simulink model "l64_clean"  (Simulink 7.9)
settings: Solver=ode45  StartTime=0.0  StopTime=1500000  RelTol=1e-3
undefined symbols (not defined in the file): pfb_coeff_gen_calc, sfp
libraries: xbsIndex_r4 x22038, casper_library_misc x643, casper_library_delays x214, xps_library x24, xbsReplacements_r4 x16, casper_library_pfbs x16, casper_library_ffts_twiddle x8, simulink x1
(part 1 of 317)
settings Model: Creator=jkocz  LastModifiedBy=jkocz
settings DebuggingCC: FcnCallInpInsideContextMsg=Use local settings  StrictBusMsg=Warning
settings GRTTargetCC: CombineOutputUpdateFcns=off  GRTInterface=on

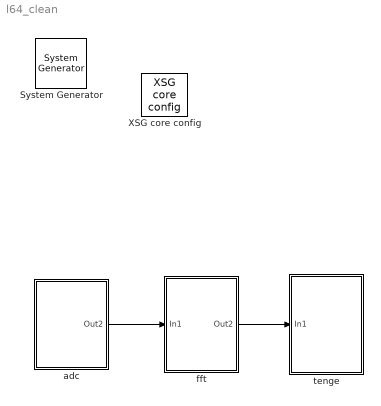
== system l64_clean ==
  " System Generator" : xbsIndex_r4/ System Generator  Tag=genX  xilinxfamily=virtex6  part=xc6vsx475t  speed=-1  package=ff1759  synthesis_tool=XST  clock_wrapper=Clock Enables  directory=./l64_clean/sysgen  proj_type=Project Navigator  Synth_file=XST Defaults  Impl_file=ISE Defaults  testbench=off  simulink_period=1  sysclk_period=5  dcm_input_clock_period=100  incr_netlist=off  trim_vbits=Everywhere in SubSystem  dbl_ovrd=According to Block Masks  core_generation=According to Block Masks  run_coregen=off  deprecated_control=off  eval_field=0  block_type=sysgen
      settings: block_version=11.4
  XSG core config : xps_library/XSG core config  Tag=xps:xsg  hw_sys=ROACH2:sx475t  clk_src=adc0_clk  ROACH_clk_src=adc0_clk  ROACH2_clk_src=adc0_clk  clk_rate=200  sample_period=1  synthesis_tool=XST
  adc : Subsystem
  fft : Subsystem
  tenge : Subsystem
  wires:
  adc/1(Out2) -> fft/1(In1)
  fft/1(Out2) -> tenge/1(In1)

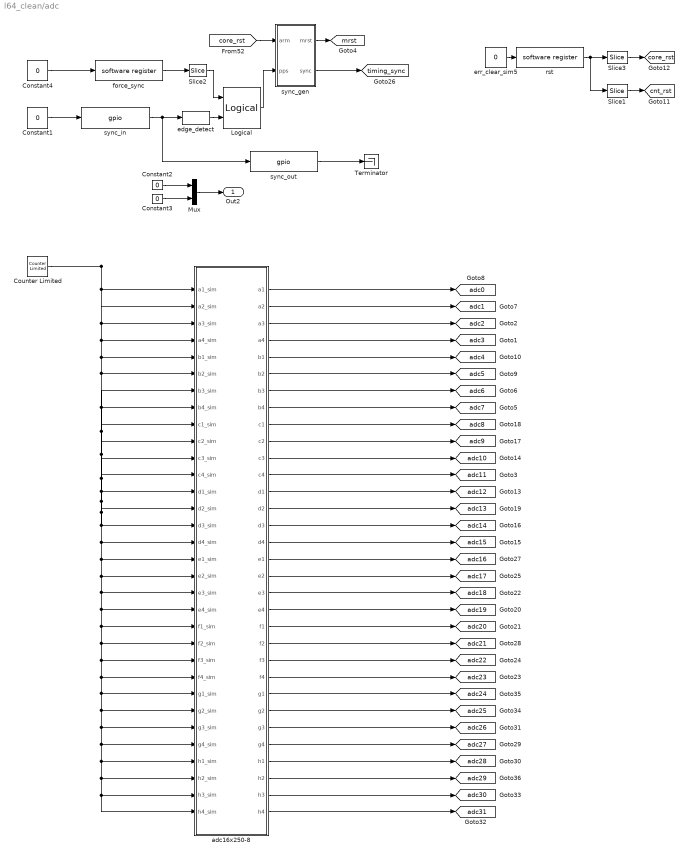
== system l64_clean/adc ==
  Constant1 : Constant  Value=0  SampleTime=1/4
  Constant2 : Constant  Value=0
  Constant3 : Constant  Value=0
  Constant4 : Constant  Value=0  SampleTime=1/4
  Counter Limited : simulink/Sources/Counter Limited  uplimit=4095  tsamp=-1
  Logical : xbsIndex_r4/Logical  logical_function=OR  inputs=2  en=off  latency=0  precision=Full  arith_type=Unsigned  n_bits=16  bin_pt=0  align_bp=on  dbl_ovrd=off  block_type=logical
  Mux : Mux  Inputs=2
  Slice1 : xbsIndex_r4/Slice  nbits=1  boolean_output=on  mode=Lower Bit Location + Width  bit1=0  base1=MSB of Input  bit0=0  base0=LSB of Input  dbl_ovrd=off  block_type=slice
      settings: block_version=10.1.3
  Slice2 : xbsIndex_r4/Slice  nbits=1  boolean_output=on  mode=Lower Bit Location + Width  bit1=0  base1=MSB of Input  bit0=0  base0=LSB of Input  dbl_ovrd=off  block_type=slice
      settings: block_version=10.1.3
  Slice3 : xbsIndex_r4/Slice  nbits=1  boolean_output=on  mode=Lower Bit Location + Width  bit1=0  base1=MSB of Input  bit0=1  base0=LSB of Input  dbl_ovrd=off  block_type=slice
      settings: block_version=10.1.3
  Terminator : Terminator
  adc16x250-8 : Subsystem  Tag=xps:adc16  AncestorBlock=xps_library/ADCs/adc16x250-8  mask(ADC16){board_count=2, roach2_rev=2, zdok_rev=1}
      mask prompts: board_count "Number of ADC16 Boards"; roach2_rev "ROACH2 Revision"; zdok_rev "ZDOK Pinout Revision"
      mask help: 16-input ADC. When using one ADC16 board, it must be in ZDOK0.
      mask init:
      | % Only board_count is needed by init function
      | adc16_init(gcb, ...
      |     'board_count', board_count);
      settings: AttributesFormatString=r2 rev%<roach2_rev>\nzdok rev%<zdok_rev>
      mask Help:
      | This is the CASPER "yellow block" for the CASPER ADC16 board.  Each ADC
      | board has four ADC chips.  Each ADC chip accepts four inputs.  The four
      | 8-bit outputs from each ADC chip are concatenated into a 32-bit signal.
      mask StyleString: popup(1|2),popup(1|2),popup(1|2)
  edge_detect : casper_library_misc/edge_detect  edge=Rising  polarity=Active High  x_in=[0 0.08 0.08 0.16 0.16 0.24 0.24 0.32 0.32 0.4]  y_in="[-1 -1  1  1  1  1  1  1  1  1]"  x_out=[0.6 0.68 0.68 0.76 0.76 0.84 0.84 0.92 0.92 1]  y_out="[-1 -1  1  1 -1 -1 -1 -1 -1 -1]"
  err_clear_sim5 : Constant  Value=0
  force_sync : xps_library/software register  Tag=xps:sw_reg  io_dir=From Processor  arith_type=Unsigned  bitwidth=32  bin_pt=0  sample_period=1  gw_name=l64_clean_adc_force_sync_user_data_out
      link override r_10gbe_demo_gbe_core_rst_user_data_out: arith_type=Unsigned  n_bits=32  bin_pt=0  period=1
      link override r_10gbe_test_cnt_rst_user_data_out: arith_type=Unsigned  n_bits=32  bin_pt=0  period=1
      link override r_10gbe_test_gbe_core_rst_user_data_out: arith_type=Unsigned  n_bits=32  bin_pt=0  period=1
      link override r_xaui_test_cnt_rst_user_data_out: arith_type=Unsigned  n_bits=32  bin_pt=0  period=1
  rst : xps_library/software register  Tag=xps:sw_reg  io_dir=From Processor  arith_type=Unsigned  bitwidth=32  bin_pt=0  sample_period=1  gw_name=l64_clean_adc_rst_user_data_out
      link override r_10gbe_demo_gbe_core_rst_user_data_out: arith_type=Unsigned  n_bits=32  bin_pt=0  period=1
      link override r_10gbe_test_cnt_rst_user_data_out: arith_type=Unsigned  n_bits=32  bin_pt=0  period=1
      link override r_10gbe_test_gbe_core_rst_user_data_out: arith_type=Unsigned  n_bits=32  bin_pt=0  period=1
      link override r_xaui_test_cnt_rst_user_data_out: arith_type=Unsigned  n_bits=32  bin_pt=0  period=1
  sync_gen : Subsystem  mask(sync_gen_hw){sync_period_bits=27}
      mask prompts: sync_period_bits "Size of sync generator counter (bits)"
      mask help:
      | This circuit implements a scheme for synchronizing a design to within an FPGA clock of an external 1 pulse-per-second signal.
      |
      | * An arm pulse will cause the sync_gen to stop issuing sync pulses.
      | * It will then ignore the next input trigger (1PPS), while sync is flushed through the DSP pipeline.
      | * It will then lock onto the next trigger (1PPS) and issue syncs thereafter every sync_period clocks.
      | * You will need a multiple of (pfb_fir taps*LCM(FFT reorder orders) *fft bins/parallel inputs). See the sync memo for further details.
  sync_in : xps_library/gpio  Tag=xps:gpio  io_group=ROACH2:sync_in  io_dir=in  arith_type=Boolean  bitwidth=1  bin_pt=0  bit_index=0  sample_period=1  use_single_ended=off  use_ddr=off  reg_iob=on  reg_clk_phase=0  termination=None
  sync_out : xps_library/gpio  Tag=xps:gpio  io_group=ROACH2:sync_out  io_dir=out  arith_type=Boolean  bitwidth=1  bin_pt=0  bit_index=0  sample_period=1  use_single_ended=off  use_ddr=off  reg_iob=on  reg_clk_phase=0  termination=None
  Out2 : Outport
  tag scope: adc0=global, adc10=global, adc11=global, adc12=global, adc13=global, adc14=global, adc15=global, adc16=global, adc17=global, adc18=global, adc19=global, adc1=global, adc20=global, adc21=global, adc22=global, adc23=global, adc24=global, adc25=global, adc26=global, adc27=global, adc28=global, adc29=global, adc2=global, adc30=global, adc31=global, adc3=global, adc4=global, adc5=global, adc6=global, adc7=global, adc8=global, adc9=global, cnt_rst=global, core_rst=global, mrst=global, timing_sync=global
  wires:
  sync_gen/1(mrst) -> [mrst]
  sync_gen/2(sync) -> [timing_sync]
  [core_rst] -> sync_gen/1(arm)
  Mux/1 -> Out2/1
  Constant2/1 -> Mux/1
  Constant3/1 -> Mux/2
  err_clear_sim5/1 -> rst/1
  rst/1 -> Slice3/1, Slice1/1
  Slice1/1 -> [cnt_rst]
  Slice3/1 -> [core_rst]
  force_sync/1 -> Slice2/1
  Constant4/1 -> force_sync/1
  adc16x250-8/1(a1) -> [adc0]
  Counter Limited/1 -> adc16x250-8/31(h3_sim), adc16x250-8/32(h4_sim), adc16x250-8/30(h2_sim), adc16x250-8/29(h1_sim), adc16x250-8/28(g4_sim), adc16x250-8/27(g3_sim), adc16x250-8/26(g2_sim), adc16x250-8/25(g1_sim), adc16x250-8/24(f4_sim), adc16x250-8/23(f3_sim), adc16x250-8/22(f2_sim), adc16x250-8/21(f1_sim), adc16x250-8/20(e4_sim), adc16x250-8/19(e3_sim), adc16x250-8/18(e2_sim), adc16x250-8/17(e1_sim), adc16x250-8/16(d4_sim), adc16x250-8/15(d3_sim), adc16x250-8/14(d2_sim), adc16x250-8/13(d1_sim), adc16x250-8/12(c4_sim), adc16x250-8/11(c3_sim), adc16x250-8/10(c2_sim), adc16x250-8/9(c1_sim), adc16x250-8/8(b4_sim), adc16x250-8/7(b3_sim), adc16x250-8/6(b2_sim), adc16x250-8/5(b1_sim), adc16x250-8/4(a4_sim), adc16x250-8/3(a3_sim), adc16x250-8/2(a2_sim), adc16x250-8/1(a1_sim)
  adc16x250-8/2(a2) -> [adc1]
  adc16x250-8/3(a3) -> [adc2]
  adc16x250-8/4(a4) -> [adc3]
  adc16x250-8/5(b1) -> [adc4]
  adc16x250-8/6(b2) -> [adc5]
  adc16x250-8/7(b3) -> [adc6]
  adc16x250-8/8(b4) -> [adc7]
  adc16x250-8/9(c1) -> [adc8]
  adc16x250-8/10(c2) -> [adc9]
  adc16x250-8/11(c3) -> [adc10]
  adc16x250-8/12(c4) -> [adc11]
  adc16x250-8/13(d1) -> [adc12]
  adc16x250-8/14(d2) -> [adc13]
  adc16x250-8/15(d3) -> [adc14]
  adc16x250-8/16(d4) -> [adc15]
  adc16x250-8/17(e1) -> [adc16]
  adc16x250-8/18(e2) -> [adc17]
  adc16x250-8/19(e3) -> [adc18]
  adc16x250-8/20(e4) -> [adc19]
  adc16x250-8/21(f1) -> [adc20]
  adc16x250-8/22(f2) -> [adc21]
  adc16x250-8/23(f3) -> [adc22]
  adc16x250-8/24(f4) -> [adc23]
  adc16x250-8/25(g1) -> [adc24]
  adc16x250-8/26(g2) -> [adc25]
  adc16x250-8/27(g3) -> [adc26]
  adc16x250-8/28(g4) -> [adc27]
  adc16x250-8/29(h1) -> [adc28]
  adc16x250-8/30(h2) -> [adc29]
  adc16x250-8/31(h3) -> [adc30]
  adc16x250-8/32(h4) -> [adc31]
  Constant1/1 -> sync_in/1
  Logical/1 -> sync_gen/2(pps)
  sync_out/1 -> Terminator/1
  edge_detect/1 -> Logical/2
  Slice2/1 -> Logical/1
  sync_in/1 -> edge_detect/1, sync_out/1

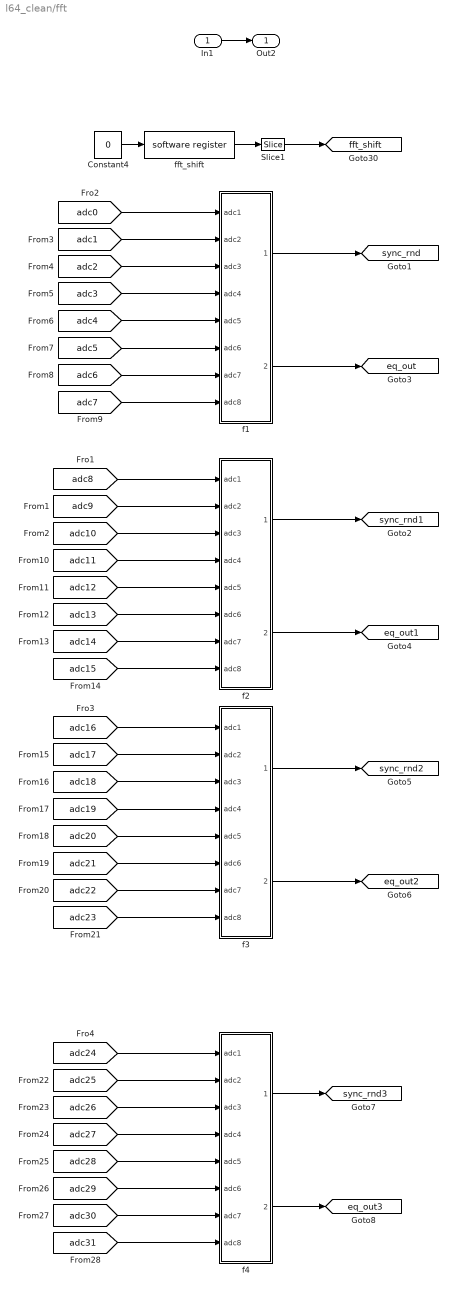
== system l64_clean/fft ==
  In1 : Inport
  Constant4 : Constant  Value=0  SampleTime=1/4
  Slice1 : xbsIndex_r4/Slice  nbits=16  boolean_output=off  mode=Lower Bit Location + Width  bit1=0  base1=MSB of Input  bit0=0  base0=LSB of Input  dbl_ovrd=off  block_type=slice
      settings: block_version=10.1.3
  f1 : Subsystem
  f2 : Subsystem
  f3 : Subsystem
  f4 : Subsystem
  fft_shift : xps_library/software register  Tag=xps:sw_reg  io_dir=From Processor  arith_type=Unsigned  bitwidth=32  bin_pt=0  sample_period=1  gw_name=l64_clean_fft_fft_shift_user_data_out
      link override r_10gbe_demo_gbe_core_rst_user_data_out: arith_type=Unsigned  n_bits=32  bin_pt=0  period=1
      link override r_10gbe_test_cnt_rst_user_data_out: arith_type=Unsigned  n_bits=32  bin_pt=0  period=1
      link override r_10gbe_test_gbe_core_rst_user_data_out: arith_type=Unsigned  n_bits=32  bin_pt=0  period=1
      link override r_xaui_test_cnt_rst_user_data_out: arith_type=Unsigned  n_bits=32  bin_pt=0  period=1
  Out2 : Outport
  tag scope: eq_out1=global, eq_out2=global, eq_out3=global, eq_out=global, fft_shift=global, sync_rnd1=global, sync_rnd2=global, sync_rnd3=global, sync_rnd=global
  wires:
  In1/1 -> Out2/1
  fft_shift/1 -> Slice1/1
  Constant4/1 -> fft_shift/1
  Slice1/1 -> [fft_shift]
  [adc0] -> f1/1(adc1)
  [adc1] -> f1/2(adc2)
  f1/1(sync_rnd) -> [sync_rnd]
  f1/2(eq_out) -> [eq_out]
  [adc2] -> f1/3(adc3)
  [adc3] -> f1/4(adc4)
  [adc4] -> f1/5(adc5)
  [adc5] -> f1/6(adc6)
  [adc6] -> f1/7(adc7)
  [adc7] -> f1/8(adc8)
  f2/1(sync_rnd) -> [sync_rnd1]
  f2/2(eq_out) -> [eq_out1]
  [adc8] -> f2/1(adc1)
  [adc9] -> f2/2(adc2)
  [adc10] -> f2/3(adc3)
  [adc11] -> f2/4(adc4)
  [adc12] -> f2/5(adc5)
  [adc13] -> f2/6(adc6)
  [adc14] -> f2/7(adc7)
  [adc15] -> f2/8(adc8)
  [adc16] -> f3/1(adc1)
  [adc17] -> f3/2(adc2)
  [adc18] -> f3/3(adc3)
  [adc19] -> f3/4(adc4)
  [adc20] -> f3/5(adc5)
  [adc21] -> f3/6(adc6)
  [adc22] -> f3/7(adc7)
  [adc23] -> f3/8(adc8)
  f3/1(sync_rnd) -> [sync_rnd2]
  f3/2(eq_out) -> [eq_out2]
  f4/1(sync_rnd) -> [sync_rnd3]
  f4/2(eq_out) -> [eq_out3]
  [adc24] -> f4/1(adc1)
  [adc25] -> f4/2(adc2)
  [adc26] -> f4/3(adc3)
  [adc27] -> f4/4(adc4)
  [adc28] -> f4/5(adc5)
  [adc29] -> f4/6(adc6)
  [adc30] -> f4/7(adc7)
  [adc31] -> f4/8(adc8)

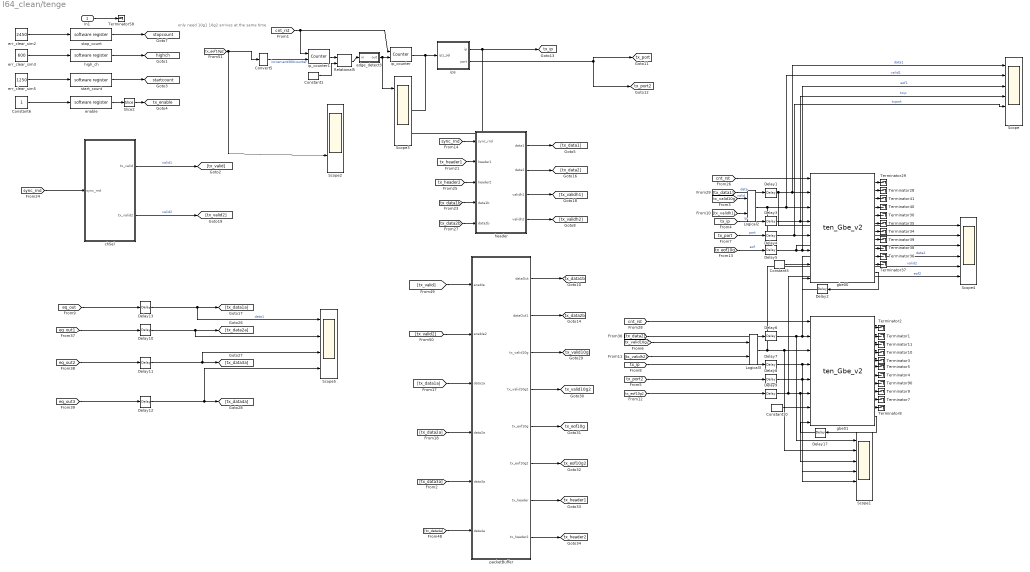
== system l64_clean/tenge ==
  note: only need 10g1
      | 10g2 arrives at the same time
  In1 : Inport
  Constant1 : xbsIndex_r4/Constant  const=1  gui_display_data_type=Fixed-point  arith_type=Unsigned  n_bits=2  bin_pt=0  preci_type=Single  exp_width=8  frac_width=24  explicit_period=on  period=1  equ=P=C  opselect=C  inp2=PCIN>>17  opr=+  inp1=P  carry=CIN  dbl_ovrd=off  block_type=constant
  Constant10 : xbsIndex_r4/Constant  const=1  gui_display_data_type=Fixed-point  arith_type=Boolean  n_bits=1  bin_pt=0  preci_type=Single  exp_width=8  frac_width=24  explicit_period=on  period=1  equ=P=C  opselect=C  inp2=PCIN>>17  opr=+  inp1=P  carry=CIN  dbl_ovrd=off  block_type=constant
  Constant3 : xbsIndex_r4/Constant  const=1  gui_display_data_type=Fixed-point  arith_type=Boolean  n_bits=1  bin_pt=0  preci_type=Single  exp_width=8  frac_width=24  explicit_period=on  period=1  equ=P=C  opselect=C  inp2=PCIN>>17  opr=+  inp1=P  carry=CIN  dbl_ovrd=off  block_type=constant
  Constant6 : Constant
  Convert5 : xbsIndex_r4/Convert  gui_display_data_type=Boolean  arith_type=Boolean  n_bits=64  bin_pt=0  float_type=Single  exp_bits=8  fraction_bits=24  quantization=Truncate  overflow=Wrap  en=off  latency=1  dbl_ovrd=off  pipeline=off  block_type=convert
      settings: block_version=11.4
  Delay{1..4,6..8,17} (8 alike) : xbsIndex_r4/Delay  rst=off  infoeditControl=Selection of Reset will increase slice count due to use of real FFs and instead of SRLs  en=off  latency=1  dbl_ovrd=off  reg_retiming=on  block_type=delay
  Delay{10..13} (4 alike) : xbsIndex_r4/Delay  rst=off  infoeditControl=Selection of Reset will increase slice count due to use of real FFs and instead of SRLs  en=off  latency=5  dbl_ovrd=off  reg_retiming=on  block_type=delay
      settings: xl_area=[0, 0, 0, 0, 0, 0, 0]
  Delay5 : xbsIndex_r4/Delay  rst=off  infoeditControl=Selection of Reset will increase slice count due to use of real FFs and instead of SRLs  en=off  latency=3  dbl_ovrd=off  reg_retiming=on  block_type=delay
  Delay9 : xbsIndex_r4/Delay  rst=off  infoeditControl=Selection of Reset will increase slice count due to use of real FFs and instead of SRLs  en=off  latency=3  dbl_ovrd=off  reg_retiming=on  block_type=delay
  Logical2 : xbsIndex_r4/Logical  logical_function=OR  inputs=2  en=off  latency=3  precision=Full  arith_type=Unsigned  n_bits=16  bin_pt=0  align_bp=on  dbl_ovrd=off  block_type=logical
      settings: block_version=11.4
  Logical3 : xbsIndex_r4/Logical  logical_function=OR  inputs=2  en=off  latency=3  precision=Full  arith_type=Unsigned  n_bits=16  bin_pt=0  align_bp=on  dbl_ovrd=off  block_type=logical
      settings: block_version=11.4
  Relational5 : xbsIndex_r4/Relational  mode=a=b  en=off  latency=1  dbl_ovrd=off  block_type=relational
      settings: xl_area=[0, 0, 0, 0, 0, 0, 0]  block_version=10.1.3
  Scope : Scope  NumInputPorts=6
      settings: TimeRange=125000  YMin=0~0~0~4012~3800.95~-1  YMax=7000~1~1~4022~4201.05~1  SaveName=ScopeData8
  Scope1 : Scope  NumInputPorts=6
      settings: YMin=0~0~0~0~0~-5  YMax=900~1~1~3~30000~5  SaveName=ScopeData9
  Scope2 : Scope  NumInputPorts=2
      settings: YMin=0~0  YMax=7000~1  SaveName=ScopeData7
  Scope3 : Scope  NumInputPorts=3
      settings: YMin=0~0~-5  YMax=7000~1~5  SaveName=ScopeData10
  Scope4 : Scope  NumInputPorts=6
      settings: TimeRange=125000  YMin=0~0~0~4012~3800.95~-1  YMax=7000~1~1~4022~4201.05~1  SaveName=ScopeData11
  Scope5 : Scope  NumInputPorts=4
      settings: YMin=0~0~-5~-5  YMax=7000~1~5~5  SaveName=ScopeData13
  Slice2 : xbsIndex_r4/Slice  nbits=1  boolean_output=off  mode=Lower Bit Location + Width  bit1=1  base1=MSB of Input  bit0=0  base0=LSB of Input  dbl_ovrd=off  block_type=slice
      settings: block_version=10.1.3
  Terminator{1..5,7..11,28..30,34..41,58,90} (23 alike) : Terminator
  chSel : Subsystem
  edge_detect3 : Subsystem  AncestorBlock=casper_library_misc/edge_detect  mask(edge_detect){edge=Rising, polarity=Active High, x_in=[0 0.08 0.08 0.16 0.16 0.24 0.24 0.32 0.32 0.4], y_in=[-1 -1  1  1  1  1  1  1  1  1], x_out=[0.6 0.68 0.68 0.76 0.76 0.84 0.84 0.92 0.92 1], y_out=[-1 -1  1  1 -1 -1 -1 -1 -1 -1]}
      mask prompts: edge "Edge Type"; polarity "Output Polarity"; x_in "Input X Positions"; y_in "Input Y Positions"; x_out "Output X Positions"; y_out "Output Y Positions"
      mask help: Output is active for one-cycle after each edge of the specified type (i.e. rising, falling or both).
      mask init:
      | edge_detect_init(gcb,...
      |     'edge',edge,...
      |     'polarity',polarity,...
      |     'x_in',x_in,...
      |     'x_out',x_out,...
      |     'y_in',y_in,...
      |     'y_out',y_out);
      | 
      | x_in_num=str2num(getfield(getfield(get_param(gcb,'UserData'),'parameters'),'x_in'));
      | x_out_num=str2num(getfield(getfield(get_param(gcb,'UserData'),'parameters'),'x_out'));
      | y_in_num=str2num(getfield(getfield(get_param(gcb,'UserData'),'parameters'),'y_in'));
      | y_out_num=str2num(getfield(getfield(get_param(gcb,'UserData'),'parameters'),'y_out'));
      settings: AttributesFormatString=|
      | rising edges
      | active high
      mask Help: Output is active for one-cycle after each edge of the specified type (i.e. rising, falling, or both).  Latency is zero.
      mask StyleString: popup(Rising|Falling|Both),popup(Active High|Active Low),edit,edit,edit,edit
      mask Display:
      | plot(x_in_num,0.5+y_in_num*0.2);
      | plot(x_out_num,0.5+y_out_num*0.2);
  enable : xps_library/software register  Tag=xps:sw_reg  io_dir=From Processor  arith_type=Unsigned  bitwidth=32  bin_pt=0  sample_period=1  gw_name=l64_clean_tenge_enable_user_data_out
      link override r_10gbe_demo_pkt_sim_run_user_data_out: arith_type=Unsigned  n_bits=32  bin_pt=0  period=1
      link override r_10gbe_test_pkt_sim_run_user_data_out: arith_type=Unsigned  n_bits=32  bin_pt=0  period=1
      link override test_pkt_buffer_10_1_p_mux_up_run_user_data_out: arith_type=Unsigned  n_bits=32  bin_pt=0  period=1
  err_clear_sim2 : Constant  Value=2450
  err_clear_sim3 : Constant  Value=600
  err_clear_sim5 : Constant  Value=1250
  gbe00 : xps_library/ten_Gbe_v2  Tag=xps:tengbe_v2  flavour=sfp+  slot=0  port_r1=0  port_r2_cx4=0  port_r2_sfpp=0  rx_dist_ram=off  large_frames=on  show_param=on  pre_emph_r2=0.74  pre_emph=3  post_emph_r2=0.18  rxeqmix_r2=7  swing_r2=940  swing=800  fab_en=off  fab_mac=hex2dec('123456789100')  fab_ip=192*(2^24) + 168*(2^16) + 5*(2^8) + 14*(2^0)  fab_udp=10002  fab_gate=192*(2^24) + 168*(2^16) + 5*(2^8) + 0  cpu_rx_en=on  cpu_tx_en=on
  gbe01 : xps_library/ten_Gbe_v2  Tag=xps:tengbe_v2  flavour=sfp+  slot=0  port_r1=0  port_r2_cx4=0  port_r2_sfpp=1  rx_dist_ram=off  large_frames=on  show_param=on  pre_emph_r2=0.74  pre_emph=3  post_emph_r2=0.18  rxeqmix_r2=7  swing_r2=940  swing=800  fab_en=off  fab_mac=hex2dec('123456789105')  fab_ip=192*(2^24) + 168*(2^16) + 6*(2^8) + 15*(2^0)  fab_udp=10002  fab_gate=192*(2^24) + 168*(2^16) + 6*(2^8) + 0  cpu_rx_en=on  cpu_tx_en=on
  header : Subsystem
  high_ch : xps_library/software register  Tag=xps:sw_reg  io_dir=From Processor  arith_type=Unsigned  bitwidth=32  bin_pt=0  sample_period=1  gw_name=l64_clean_tenge_high_ch_user_data_out
      link override r_10gbe_demo_dest_ip_user_data_out: arith_type=Unsigned  n_bits=32  bin_pt=0  period=1
      link override r_10gbe_test_dest_ip_user_data_out: arith_type=Unsigned  n_bits=32  bin_pt=0  period=1
      link override r_xaui_test_cnt_rst_user_data_out: arith_type=Unsigned  n_bits=32  bin_pt=0  period=1
  ip_counter : xbsIndex_r4/Counter  cnt_type=Free Running  cnt_to=3  operation=Up  start_count=0  cnt_by_val=1  arith_type=Unsigned  n_bits=2  bin_pt=0  load_pin=off  rst=on  en=on  explicit_period=on  period=1  dbl_ovrd=off  use_behavioral_HDL=off  implementation=Fabric  block_type=counter
      settings: block_version=11.4
  ip_counter1 : xbsIndex_r4/Counter  cnt_type=Free Running  cnt_to=0  operation=Up  start_count=1  cnt_by_val=1  arith_type=Unsigned  n_bits=1  bin_pt=0  load_pin=off  rst=on  en=on  explicit_period=on  period=1  dbl_ovrd=off  use_behavioral_HDL=off  implementation=Fabric  block_type=counter
      settings: block_version=11.4
  ips : Subsystem
  packetBuffer : Subsystem
  start_count : xps_library/software register  Tag=xps:sw_reg  io_dir=From Processor  arith_type=Unsigned  bitwidth=32  bin_pt=0  sample_period=1  gw_name=l64_clean_tenge_start_count_user_data_out
      link override r_10gbe_demo_dest_ip_user_data_out: arith_type=Unsigned  n_bits=32  bin_pt=0  period=1
      link override r_10gbe_test_dest_ip_user_data_out: arith_type=Unsigned  n_bits=32  bin_pt=0  period=1
      link override r_xaui_test_cnt_rst_user_data_out: arith_type=Unsigned  n_bits=32  bin_pt=0  period=1
  stop_count : xps_library/software register  Tag=xps:sw_reg  io_dir=From Processor  arith_type=Unsigned  bitwidth=32  bin_pt=0  sample_period=1  gw_name=l64_clean_tenge_stop_count_user_data_out
      link override leda_64_sim_tenge_stop_count_user_data_out: arith_type=Unsigned  n_bits=32  bin_pt=0  period=1
      link override r_10gbe_demo_dest_ip_user_data_out: arith_type=Unsigned  n_bits=32  bin_pt=0  period=1
      link override r_10gbe_test_dest_ip_user_data_out: arith_type=Unsigned  n_bits=32  bin_pt=0  period=1
      link override r_xaui_test_cnt_rst_user_data_out: arith_type=Unsigned  n_bits=32  bin_pt=0  period=1
  tag scope: highch=global, startcount=global, stopcount=global, tx_data1b=global, tx_data2b=global, tx_enable=global, tx_eof10g2=global, tx_eof10g=global, tx_header1=global, tx_header2=global, tx_ip=global, tx_port2=global, tx_port=global, tx_valid10g2=global, tx_valid10g=global
  wires:
  In1/1 -> Terminator58/1
  err_clear_sim2/1 -> stop_count/1
  stop_count/1 -> [stopcount]
  err_clear_sim3/1 -> high_ch/1
  high_ch/1 -> [highch]
  err_clear_sim5/1 -> start_count/1
  start_count/1 -> [startcount]
  enable/1 -> Slice2/1
  Constant6/1 -> enable/1
  Slice2/1 -> [tx_enable]
  [sync_rnd] -> chSel/1(sync_rnd)
  gbe00/12 -> Delay2/1
  Delay2/1 -> gbe00/8
  gbe00/5 -> Terminator30/1
  gbe00/4 -> Terminator40/1
  gbe00/9 -> Terminator38/1
  gbe00/1 -> Terminator29/1
  gbe00/2 -> Terminator28/1
  gbe00/3 -> Terminator41/1
  gbe00/6 -> Terminator35/1
  gbe00/7 -> Terminator34/1
  gbe00/8 -> Terminator39/1
  gbe00/10 -> Terminator36/1
  gbe00/11 -> Terminator37/1
  [cnt_rst] -> gbe00/1
  Constant10/1 -> gbe01/7
  gbe01/12 -> Delay17/1
  Delay17/1 -> gbe01/8
  gbe01/5 -> Terminator3/1
  gbe01/4 -> Terminator10/1
  gbe01/9 -> Terminator9/1
  gbe01/1 -> Terminator2/1
  gbe01/2 -> Terminator1/1
  gbe01/3 -> Terminator11/1
  gbe01/6 -> Terminator5/1
  gbe01/7 -> Terminator4/1
  gbe01/8 -> Terminator90/1
  gbe01/10 -> Terminator7/1
  gbe01/11 -> Terminator8/1
  [cnt_rst] -> gbe01/1
  Constant3/1 -> gbe00/7
  ips/1(ip) -> [tx_ip], Scope3/3
  ip_counter/1 -> ips/1(ip1_sel), Scope3/2
  [cnt_rst] -> ip_counter/1, ip_counter1/1
  chSel/1(tx_valid) -> [tx_valid]  "valid1"
  [tx_ip] -> Delay3/1  "ip"
  [tx_port] -> Delay4/1  "port"
  [tx_ip] -> Delay7/1
  [tx_port2] -> Delay8/1
  chSel/2(tx_valid2) -> [tx_valid2]  "valid2"
  [tx_eof10g2] -> Delay9/1
  [tx_eof10g] -> Delay5/1  "eof"
  packetBuffer/1(dataOut) -> [tx_data1b]
  [tx_data1] -> Delay1/1  "data"
  [tx_data2] -> Delay6/1
  packetBuffer/2(dataOut1) -> [tx_data2b]
  [tx_valid] -> packetBuffer/1(enable)
  packetBuffer/3(tx_valid10g) -> [tx_valid10g]
  packetBuffer/4(tx_valid10g2) -> [tx_valid10g2]
  packetBuffer/5(tx_eof10g) -> [tx_eof10g]
  packetBuffer/6(tx_eof10g2) -> [tx_eof10g2]
  [tx_valid2] -> packetBuffer/2(enable2)
  [tx_data1a] -> packetBuffer/3(data1a)
  [tx_data2a] -> packetBuffer/4(data2a)
  [tx_data3a] -> packetBuffer/5(data3a)
  [tx_data4a] -> packetBuffer/6(data4a)
  [tx_eof10g2] -> Scope2/2, Convert5/1
  packetBuffer/7(tx_header) -> [tx_header1]
  packetBuffer/8(tx_header2) -> [tx_header2]
  [tx_header2] -> header/3(header2)
  [tx_header1] -> header/2(header1)
  [sync_rnd] -> header/1(sync_rnd)
  [tx_data1b] -> header/4(data1b)
  [tx_data2b] -> header/5(data2b)
  header/1(data1) -> [tx_data1]
  header/2(data2) -> [tx_data2]
  [tx_valid10g] -> Logical2/1  "valid"
  Logical2/1 -> gbe00/3, Scope/2, Scope4/2  "valid1"
  [tx_valid10g2] -> Logical3/1
  Logical3/1 -> gbe01/3, Scope1/2, Scope4/5  "valid2"
  header/3(validh1) -> [tx_validh1]
  header/4(validh2) -> [tx_validh2]
  [tx_validh1] -> Logical2/2
  [tx_validh2] -> Logical3/2
  Delay1/1 -> gbe00/2, Scope/1, Scope4/1  "data1"
  Delay3/1 -> gbe00/4, Scope/4  "txip"
  Delay4/1 -> gbe00/5, Scope/5  "txport"
  Delay5/1 -> gbe00/6, Scope/3, Scope4/3  "eof1"
  Delay6/1 -> Scope1/1, gbe01/2, Scope4/4  "data2"
  Delay7/1 -> gbe01/4, Scope1/4
  Delay8/1 -> gbe01/5, Scope1/5
  Delay9/1 -> gbe01/6, Scope1/3, Scope4/6  "eof2"
  [eq_out1] -> Delay10/1
  Delay10/1 -> [tx_data2a], Scope5/2
  [eq_out2] -> Delay11/1
  Delay11/1 -> [tx_data3a], Scope5/3
  [eq_out3] -> Delay12/1
  Delay12/1 -> [tx_data4a], Scope5/4
  [eq_out] -> Delay13/1
  Delay13/1 -> [tx_data1a], Scope5/1  "data1"
  Convert5/1 -> ip_counter1/2  "increment300counter"
  edge_detect3/1(out) -> ip_counter/2, Scope3/1
  ip_counter1/1 -> Relational5/1
  Relational5/1 -> edge_detect3/1(in)
  Constant1/1 -> Relational5/2
  ips/2(port) -> [tx_port], [tx_port2]

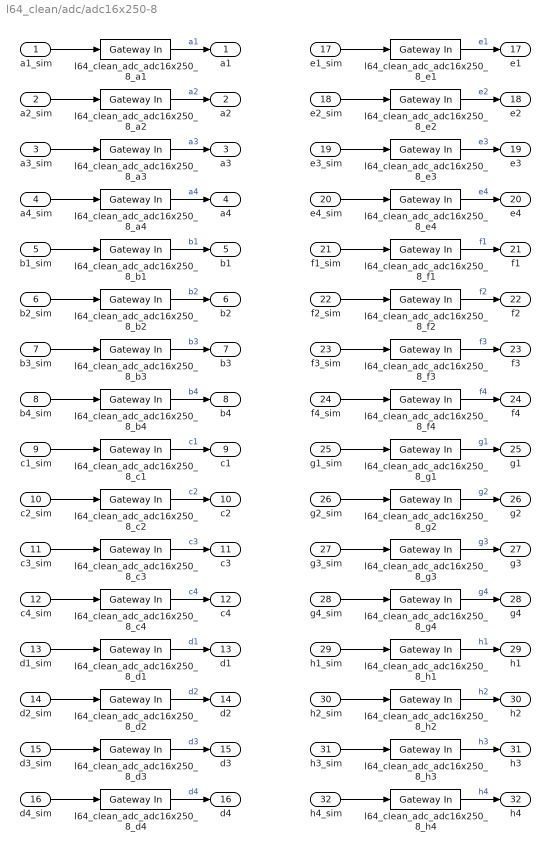
== system l64_clean/adc/adc16x250-8 ==
  a1_sim : Inport
  a2_sim : Inport  Port=2
  a3_sim : Inport  Port=3
  a4_sim : Inport  Port=4
  b1_sim : Inport  Port=5
  b2_sim : Inport  Port=6
  b3_sim : Inport  Port=7
  b4_sim : Inport  Port=8
  c1_sim : Inport  Port=9
  c2_sim : Inport  Port=10
  c3_sim : Inport  Port=11
  c4_sim : Inport  Port=12
  d1_sim : Inport  Port=13
  d2_sim : Inport  Port=14
  d3_sim : Inport  Port=15
  d4_sim : Inport  Port=16
  e1_sim : Inport  Port=17
  e2_sim : Inport  Port=18
  e3_sim : Inport  Port=19
  e4_sim : Inport  Port=20
  f1_sim : Inport  Port=21
  f2_sim : Inport  Port=22
  f3_sim : Inport  Port=23
  f4_sim : Inport  Port=24
  g1_sim : Inport  Port=25
  g2_sim : Inport  Port=26
  g3_sim : Inport  Port=27
  g4_sim : Inport  Port=28
  h1_sim : Inport  Port=29
  h2_sim : Inport  Port=30
  h3_sim : Inport  Port=31
  h4_sim : Inport  Port=32
  l64_clean_adc_adc16x250_8_a{1..4} (4 alike) : xbsIndex_r4/Gateway In  gui_display_data_type=Fixed-point  arith_type="Signed  (2's comp)"  n_bits=8  bin_pt=7  preci_type=Single  exp_width=8  frac_width=24  quantization="Round  (unbiased: +/- Inf)"  overflow=Saturate  period=1  dbl_ovrd=off  timing_constraint=None  locs_specified=off  LOCs={}  UseAsADC=off  ADCChannel='1'  inherit_from_input=off  hdl_port=on  block_type=gatewayin
  l64_clean_adc_adc16x250_8_b{1..4} (4 alike) : xbsIndex_r4/Gateway In  gui_display_data_type=Fixed-point  arith_type="Signed  (2's comp)"  n_bits=8  bin_pt=7  preci_type=Single  exp_width=8  frac_width=24  quantization="Round  (unbiased: +/- Inf)"  overflow=Saturate  period=1  dbl_ovrd=off  timing_constraint=None  locs_specified=off  LOCs={}  UseAsADC=off  ADCChannel='1'  inherit_from_input=off  hdl_port=on  block_type=gatewayin
  l64_clean_adc_adc16x250_8_c{1..4} (4 alike) : xbsIndex_r4/Gateway In  gui_display_data_type=Fixed-point  arith_type="Signed  (2's comp)"  n_bits=8  bin_pt=7  preci_type=Single  exp_width=8  frac_width=24  quantization="Round  (unbiased: +/- Inf)"  overflow=Saturate  period=1  dbl_ovrd=off  timing_constraint=None  locs_specified=off  LOCs={}  UseAsADC=off  ADCChannel='1'  inherit_from_input=off  hdl_port=on  block_type=gatewayin
  l64_clean_adc_adc16x250_8_d{1..4} (4 alike) : xbsIndex_r4/Gateway In  gui_display_data_type=Fixed-point  arith_type="Signed  (2's comp)"  n_bits=8  bin_pt=7  preci_type=Single  exp_width=8  frac_width=24  quantization="Round  (unbiased: +/- Inf)"  overflow=Saturate  period=1  dbl_ovrd=off  timing_constraint=None  locs_specified=off  LOCs={}  UseAsADC=off  ADCChannel='1'  inherit_from_input=off  hdl_port=on  block_type=gatewayin
  l64_clean_adc_adc16x250_8_e{1..4} (4 alike) : xbsIndex_r4/Gateway In  gui_display_data_type=Fixed-point  arith_type="Signed  (2's comp)"  n_bits=8  bin_pt=7  preci_type=Single  exp_width=8  frac_width=24  quantization="Round  (unbiased: +/- Inf)"  overflow=Saturate  period=1  dbl_ovrd=off  timing_constraint=None  locs_specified=off  LOCs={}  UseAsADC=off  ADCChannel='1'  inherit_from_input=off  hdl_port=on  block_type=gatewayin
  l64_clean_adc_adc16x250_8_f{1..4} (4 alike) : xbsIndex_r4/Gateway In  gui_display_data_type=Fixed-point  arith_type="Signed  (2's comp)"  n_bits=8  bin_pt=7  preci_type=Single  exp_width=8  frac_width=24  quantization="Round  (unbiased: +/- Inf)"  overflow=Saturate  period=1  dbl_ovrd=off  timing_constraint=None  locs_specified=off  LOCs={}  UseAsADC=off  ADCChannel='1'  inherit_from_input=off  hdl_port=on  block_type=gatewayin
  l64_clean_adc_adc16x250_8_g{1..4} (4 alike) : xbsIndex_r4/Gateway In  gui_display_data_type=Fixed-point  arith_type="Signed  (2's comp)"  n_bits=8  bin_pt=7  preci_type=Single  exp_width=8  frac_width=24  quantization="Round  (unbiased: +/- Inf)"  overflow=Saturate  period=1  dbl_ovrd=off  timing_constraint=None  locs_specified=off  LOCs={}  UseAsADC=off  ADCChannel='1'  inherit_from_input=off  hdl_port=on  block_type=gatewayin
  l64_clean_adc_adc16x250_8_h{1..4} (4 alike) : xbsIndex_r4/Gateway In  gui_display_data_type=Fixed-point  arith_type="Signed  (2's comp)"  n_bits=8  bin_pt=7  preci_type=Single  exp_width=8  frac_width=24  quantization="Round  (unbiased: +/- Inf)"  overflow=Saturate  period=1  dbl_ovrd=off  timing_constraint=None  locs_specified=off  LOCs={}  UseAsADC=off  ADCChannel='1'  inherit_from_input=off  hdl_port=on  block_type=gatewayin
  a1 : Outport
  a2 : Outport  Port=2
  a3 : Outport  Port=3
  a4 : Outport  Port=4
  b1 : Outport  Port=5
  b2 : Outport  Port=6
  b3 : Outport  Port=7
  b4 : Outport  Port=8
  c1 : Outport  Port=9
  c2 : Outport  Port=10
  c3 : Outport  Port=11
  c4 : Outport  Port=12
  d1 : Outport  Port=13
  d2 : Outport  Port=14
  d3 : Outport  Port=15
  d4 : Outport  Port=16
  e1 : Outport  Port=17
  e2 : Outport  Port=18
  e3 : Outport  Port=19
  e4 : Outport  Port=20
  f1 : Outport  Port=21
  f2 : Outport  Port=22
  f3 : Outport  Port=23
  f4 : Outport  Port=24
  g1 : Outport  Port=25
  g2 : Outport  Port=26
  g3 : Outport  Port=27
  g4 : Outport  Port=28
  h1 : Outport  Port=29
  h2 : Outport  Port=30
  h3 : Outport  Port=31
  h4 : Outport  Port=32
  wires:
  a1_sim/1 -> l64_clean_adc_adc16x250_8_a1/1
  l64_clean_adc_adc16x250_8_a1/1 -> a1/1  "a1"
  a2_sim/1 -> l64_clean_adc_adc16x250_8_a2/1
  l64_clean_adc_adc16x250_8_a2/1 -> a2/1  "a2"
  a3_sim/1 -> l64_clean_adc_adc16x250_8_a3/1
  l64_clean_adc_adc16x250_8_a3/1 -> a3/1  "a3"
  a4_sim/1 -> l64_clean_adc_adc16x250_8_a4/1
  l64_clean_adc_adc16x250_8_a4/1 -> a4/1  "a4"
  b1_sim/1 -> l64_clean_adc_adc16x250_8_b1/1
  l64_clean_adc_adc16x250_8_b1/1 -> b1/1  "b1"
  b2_sim/1 -> l64_clean_adc_adc16x250_8_b2/1
  l64_clean_adc_adc16x250_8_b2/1 -> b2/1  "b2"
  b3_sim/1 -> l64_clean_adc_adc16x250_8_b3/1
  l64_clean_adc_adc16x250_8_b3/1 -> b3/1  "b3"
  b4_sim/1 -> l64_clean_adc_adc16x250_8_b4/1
  l64_clean_adc_adc16x250_8_b4/1 -> b4/1  "b4"
  c1_sim/1 -> l64_clean_adc_adc16x250_8_c1/1
  l64_clean_adc_adc16x250_8_c1/1 -> c1/1  "c1"
  c2_sim/1 -> l64_clean_adc_adc16x250_8_c2/1
  l64_clean_adc_adc16x250_8_c2/1 -> c2/1  "c2"
  c3_sim/1 -> l64_clean_adc_adc16x250_8_c3/1
  l64_clean_adc_adc16x250_8_c3/1 -> c3/1  "c3"
  c4_sim/1 -> l64_clean_adc_adc16x250_8_c4/1
  l64_clean_adc_adc16x250_8_c4/1 -> c4/1  "c4"
  d1_sim/1 -> l64_clean_adc_adc16x250_8_d1/1
  l64_clean_adc_adc16x250_8_d1/1 -> d1/1  "d1"
  d2_sim/1 -> l64_clean_adc_adc16x250_8_d2/1
  l64_clean_adc_adc16x250_8_d2/1 -> d2/1  "d2"
  d3_sim/1 -> l64_clean_adc_adc16x250_8_d3/1
  l64_clean_adc_adc16x250_8_d3/1 -> d3/1  "d3"
  d4_sim/1 -> l64_clean_adc_adc16x250_8_d4/1
  l64_clean_adc_adc16x250_8_d4/1 -> d4/1  "d4"
  e1_sim/1 -> l64_clean_adc_adc16x250_8_e1/1
  l64_clean_adc_adc16x250_8_e1/1 -> e1/1  "e1"
  e2_sim/1 -> l64_clean_adc_adc16x250_8_e2/1
  l64_clean_adc_adc16x250_8_e2/1 -> e2/1  "e2"
  e3_sim/1 -> l64_clean_adc_adc16x250_8_e3/1
  l64_clean_adc_adc16x250_8_e3/1 -> e3/1  "e3"
  e4_sim/1 -> l64_clean_adc_adc16x250_8_e4/1
  l64_clean_adc_adc16x250_8_e4/1 -> e4/1  "e4"
  f1_sim/1 -> l64_clean_adc_adc16x250_8_f1/1
  l64_clean_adc_adc16x250_8_f1/1 -> f1/1  "f1"
  f2_sim/1 -> l64_clean_adc_adc16x250_8_f2/1
  l64_clean_adc_adc16x250_8_f2/1 -> f2/1  "f2"
  f3_sim/1 -> l64_clean_adc_adc16x250_8_f3/1
  l64_clean_adc_adc16x250_8_f3/1 -> f3/1  "f3"
  f4_sim/1 -> l64_clean_adc_adc16x250_8_f4/1
  l64_clean_adc_adc16x250_8_f4/1 -> f4/1  "f4"
  g1_sim/1 -> l64_clean_adc_adc16x250_8_g1/1
  l64_clean_adc_adc16x250_8_g1/1 -> g1/1  "g1"
  g2_sim/1 -> l64_clean_adc_adc16x250_8_g2/1
  l64_clean_adc_adc16x250_8_g2/1 -> g2/1  "g2"
  g3_sim/1 -> l64_clean_adc_adc16x250_8_g3/1
  l64_clean_adc_adc16x250_8_g3/1 -> g3/1  "g3"
  g4_sim/1 -> l64_clean_adc_adc16x250_8_g4/1
  l64_clean_adc_adc16x250_8_g4/1 -> g4/1  "g4"
  h1_sim/1 -> l64_clean_adc_adc16x250_8_h1/1
  l64_clean_adc_adc16x250_8_h1/1 -> h1/1  "h1"
  h2_sim/1 -> l64_clean_adc_adc16x250_8_h2/1
  l64_clean_adc_adc16x250_8_h2/1 -> h2/1  "h2"
  h3_sim/1 -> l64_clean_adc_adc16x250_8_h3/1
  l64_clean_adc_adc16x250_8_h3/1 -> h3/1  "h3"
  h4_sim/1 -> l64_clean_adc_adc16x250_8_h4/1
  l64_clean_adc_adc16x250_8_h4/1 -> h4/1  "h4"

== system l64_clean/adc/sync_gen ==
  arm : Inport
  pps : Inport  Port=2
  Constant : xbsIndex_r4/Constant  const=2^sync_period_bits  gui_display_data_type=Fixed-point  arith_type=Unsigned  n_bits=sync_period_bits+1  bin_pt=0  preci_type=Single  exp_width=8  frac_width=24  explicit_period=on  period=1  equ=P=C  opselect=C  inp2=PCIN>>17  opr=+  inp1=P  carry=CIN  dbl_ovrd=off  block_type=constant
  Delay1 : xbsIndex_r4/Delay  rst=off  infoeditControl=Selection of Reset will increase slice count due to use of real FFs and instead of SRLs  en=off  latency=1  dbl_ovrd=off  reg_retiming=on  block_type=delay
  Delay2 : xbsIndex_r4/Delay  rst=off  infoeditControl=Selection of Reset will increase slice count due to use of real FFs and instead of SRLs  en=off  latency=1  dbl_ovrd=off  reg_retiming=on  block_type=delay
  rst_gen : Subsystem  mask{reset_period=1}
      mask prompts: reset_period "Min period of reset (pps pulses)"
      mask init:
      | %reset_period = eval(get_param(gcb,'reset_period'))
      | %bits = ceil(log2(reset_period))
      | %set_param([gcb,'/Counter'],'n_bits',num2str(bits));
      | %set_param([gcb,'/Counter'],'start_count', num2str(reset_period))
      | %set_param([gcb,'/Constant'],'n_bits',num2str(bits));
  sync_gen : Subsystem  mask{sync_gen_bits=sync_period_bits+1}
      mask prompts: sync_gen_bits "Size of sync generator counter"
  mrst : Outport
  sync : Outport  Port=2
  wires:
  arm/1 -> rst_gen/1(arm)
  pps/1 -> rst_gen/2(sync_in)
  sync_gen/1(sync) -> Delay2/1
  rst_gen/1(mrst) -> sync_gen/1(rst), Delay1/1
  Delay1/1 -> mrst/1
  Delay2/1 -> sync/1
  Constant/1 -> sync_gen/2(sync_period)
